simulink library "Renault_Library"  (Simulink 8.2)
settings: Solver=ode3  StartTime=0.0  StopTime=10.0  FixedStep=0.020  RelTol=1e-3
undefined symbols (not defined in the file or in its folder): ABC, AssystemProposal, b, chkval, CoeffA_C, CoeffB_C, CoeffMean_C, ConfTime_C, DiagDelay_C, DividePercent, FailureConfActive_C, FallingEdgeDuration_C, HighLevelThresholdVoltage_C, HighLevelVoltage_C, HighLevelVoltageTunable_C, InitValue_C, InputVoltRangeMax_C, InputVoltRangeMin_C, LowActiveContact_C, LowLevelThresholdVoltage_C, LowLevelVoltage_C, MaxOperatingVoltage_C, MinOperatingVoltage_C, mV2V_conversion, OUT1, OutOfRangeHigh_C, OutOfRangeLow_C, PinSupplySaturationMax, PinSupplySaturationMin, Res1Value_C, Res2Value_C, Res3Value_C, RisingEdgeDuration_C, RSA_CANSignalFailMonitoring, SampleTime_C, service14Table, service19Table, service22Table, service24Table, service28Table, service2ETable, service2FTable, service31Table, service87Table, SizeXStateDID2FTable, SizeXTable14, SizeXTable19, SizeXTable22, SizeXTable24, SizeXTable28, SizeXTable2E, SizeXTable2F, SizeXTable31, SizeXTable87, SizeYStateDID2FTable, SizeYTable14, SizeYTable19, SizeYTable22, SizeYTable24, SizeYTable28 ... (+22)
undefined symbols (not defined in the file): BatteryVoltage_ActivationThreshold_C, FrameStatus_AbsentValue_C, FrameStatus_ConfAbsentValue_C, MemThreshold_C, NAMEOFTHEMODEL_SAMPLE_TIME_CST, NbAbs1_C, NbConfAbs1_C, NbConfPres1_C, NbPres1_C, NOMDUSIGNAL_FaultConfTime_C, NOMDUSIGNAL_FaultRehabTime_C, NOMDUSIGNAL_switchvalue_C, NOMDUSIGNAL_unavailablevalue_C, PeriodFrame1_C, ResetTime_C, WakeUpTime_C
libraries: Renault_Library x112, simulink x75
(part 6 of 32)

== system Renault_Library/Services ECU/EEPROM ==
  note: EEPROM
  RSA_UpdateEEPROMData : Subsystem  Tag=[LIB]  mask(RSA_UpdateEEPROMData){}
      mask help:
      | Manage EEPROM data in model.
      |
      | Version : 01.00
      mask Help:
      | Manage EEPROM data in model.
      | 
      | Version : 01.00
      mask Display:
      | disp('EEPROM Management')
      | port_label('Input',1,'MODEL_EEPROM_DATA')
      | port_label('Input',2,'DATA_STORE_READ')
      | port_label('Output',1,'DATA_STORE_WRITE')

== system Renault_Library/Services ECU/HW Inputs ==
  note: DEFINED INPUTS
  note: FULLY TUNABLE INPUTS
  RSA_CHA8PRO : Subsystem  Tag=[LIB]  mask(RSA_CHA8PRO){HighLevelOutput_C=1, InitValue_C=0, SampleTime_C=1, Tstab_C=30}
      mask prompts: HighLevelOutput_C "State of output relay when high voltage (1 for active high, 0 for active low)"; InitValue_C "Initial Value of Output"; SampleTime_C "Ts: insert a sample time (ms)"; Tstab_C "Tstab : insert a stabilization waiting time (ms)"
      mask help:
      | CHA.8.PRO:
      | Input for active HIGH contact with medium current switch. Contact has a serial resistive protection.
      | Min Operating Voltage = 8 V
      | Max Operating Voltage = 16 V
      | High level input voltage >= 5.5V
      | Low level input voltage <= 2.5V
      | Unit of ports for voltage are in millivolt (mV)
      |
      | Version : 01.01
      mask init:
      | MinOperatingVoltage_C=8000;
      | MaxOperatingVoltage_C=16000; 
      | LowLevelThresholdVoltage_C=2500; 
      | HighLevelThresholdVoltage_C=5500; 
      | LowLevelOutput_C= not(HighLevelOutput_C);
      mask Help:
      | CHA.8.PRO:
      | Input for active HIGH contact with medium current switch. Contact has a serial resistive protection.
      | Min Operating Voltage = 8 V
      | Max Operating Voltage = 16 V
      | High level input voltage >= 5.5V
      | Low level input voltage <= 2.5V
      | Unit of ports for voltage are in millivolt (mV)
      | 
      | Version : 01.01
      mask Display: disp('CHA_8_PRO')
  RSA_CHB6 : Subsystem  Tag=[LIB]  mask(RSA_CHB6){HighLevelOutput_C=1, InitValue_C=0, SampleTime_C=1, Tstab_C=30}
      mask prompts: HighLevelOutput_C "State of output relay when high voltage (1 for active high, 0 for active low)"; InitValue_C "Initial value of Output"; SampleTime_C "Ts: insert a sample time (ms)"; Tstab_C "Tstab : insert a stabilization waiting time (ms)"
      mask help:
      | CHB.6:
      | Input for active HIGH contact shared between several consumers
      | Min Operating Voltage = 6 V
      | Max Operating Voltage = 16V
      | High level input voltage >= 5.5V
      | Low level input voltage <= 2.5V
      | Unit of ports for voltage are in millivolt (mV)
      |
      | Version : 01.01
      mask init:
      | MinOperatingVoltage_C=6000;%Min Operating Voltage. Range for which the considered input hardware stage should work correctly (mV)
      | MaxOperatingVoltage_C=16000; %Max Operating Voltage. Upper limit of range for which the considered input hardware stage should work correctly (mV)(normally 16V)
      | LowLevelThresholdVoltage_C=2500; %Low Level Threshold Voltage (mV)
      | HighLevelThresholdVoltage_C=5500; %High Level Threshold Voltage (mV)
      | 
      | LowLevelOutput_C= not(HighLevelOutput_C);
      | 
      | % InitValue_C=0; %Value of initial value when enabling
      mask Help:
      | CHB.6:
      | Input for active HIGH contact shared between several consumers
      | Min Operating Voltage = 6 V
      | Max Operating Voltage = 16V
      | High level input voltage >= 5.5V
      | Low level input voltage <= 2.5V
      | Unit of ports for voltage are in millivolt (mV)
      | 
      | Version : 01.01
      mask Display: disp('CHB_6')
  RSA_CHB6DAS : Subsystem  Tag=[LIB]  mask(RSA_CHB6DAS){HighLevelOutput_C=1, InitValue_C=0, SampleTime_C=1, Tstab_C=30}
      mask prompts: HighLevelOutput_C "State of output relay when high voltage (1 for active high, 0 for active low)"; InitValue_C "Initial value of Output"; SampleTime_C "Ts: insert a sample time (ms)"; Tstab_C "Tstab : insert a stabilization waiting time (ms)"
      mask help:
      | CHB.6.DAS:
      | Input for active HIGH contact with medium current switch. Signal shared between several consumers.
      | Min Operating Voltage = 6 V
      | Max Operating Voltage = 16V
      | High level input voltage >= 5.5V
      | Low level input voltage <= 2.5V
      | Unit of ports for voltage are in millivolt (mV)
      |
      | Version : 01.01
      mask init:
      | MinOperatingVoltage_C=6000;
      | MaxOperatingVoltage_C=16000; 
      | LowLevelThresholdVoltage_C=2500; 
      | HighLevelThresholdVoltage_C=5500; 
      | LowLevelOutput_C= not(HighLevelOutput_C);
      mask Help:
      | CHB.6.DAS:
      | Input for active HIGH contact with medium current switch. Signal shared between several consumers.
      | Min Operating Voltage = 6 V
      | Max Operating Voltage = 16V
      | High level input voltage >= 5.5V
      | Low level input voltage <= 2.5V
      | Unit of ports for voltage are in millivolt (mV)
      | 
      | Version : 01.01
      mask Display: disp('CHB_6_DAS')
  RSA_CHXX : Subsystem  Tag=[LIB]  mask(RSA_CHX.X){MinOperatingVoltage_C=0, MaxOperatingVoltage_C=0, LowLevelThresholdVoltage_C=0, HighLevelThresholdVoltage_C=0, HighLevelOutput_C=1, InitValue_C=0, SampleTime_C=1, Tstab_C=30}
      mask prompts: MinOperatingVoltage_C "Min Operating Voltage. Range for which the considered input hardware stage should work correctly (mV)"; MaxOperatingVoltage_C "Max Operating Voltage. Upper limit of range for which the considered input hardware stage should work correctly (mV)(normally 16V)"; LowLevelThresholdVoltage_C "Low Level Threshold Voltage (mV)"; HighLevelThresholdVoltage_C "High Level Threshold Voltage (mV)"; HighLevelOutput_C "State of output relay when high voltage (1 for active high, 0 for active low)"; InitValue_C "Initial value of Output"; SampleTime_C "Ts: insert a sample time (ms)"; Tstab_C "Tstab : insert a stabilization waiting time (ms)"
      mask help:
      | CHX.X:
      | Input for active HIGH contact
      | Unit of ports for voltage are in millivolt (mV)
      |
      | Version : 01.01
      mask init:
      | LowLevelOutput_C= not(HighLevelOutput_C);% InitValue_C=0; %Value of initial value when enabling
      mask Help:
      | CHX.X:
      | Input for active HIGH contact
      | Unit of ports for voltage are in millivolt (mV)
      | 
      | Version : 01.01
      mask Display: disp('CHX.X')
  RSA_CLA8LOCK : Subsystem  Tag=[LIB]  mask(RSA_CLA8LOCK){InitValue_C=0, Tstab_C=30, ConfTime_C=20, SampleTime_C=1, VonMax_C=4500, VonMin_C=500}
      mask prompts: InitValue_C "Initial Value of Output"; Tstab_C "Tstab : insert a stabilization waiting time (SoftwareFiltering) (ms)"; ConfTime_C "ConfTime : Confirmation Time in case of Failure (ms)"; SampleTime_C "SampleTime : SampleTime of block (ms)"; VonMax_C "Threshold (mV) above which a Resistive SC- failure is declared : Von_max"; VonMin_C "Threshold (mV) below which an SC- failure is declared :Von_min"
      mask help:
      | CLA.8.LOCK: Analog active low input with Interlock Switch.
      |
      | ECU input, active on low state. Link to one consumer.
      | Min Operating Voltage = 8 V
      | Max Operating Voltage = 16 V
      | Unit of ports for voltage are in millivolt (mV)
      |
      | Diagnosis:
      | Three failure states (SC+, SC-, OC), and two normal states (OKON and OKOFF) are possible.
      | The analogue thresholds to define these different states must take into account the battery voltage range.
      |
      |     State           SW Status               Diagnostic States
      |      4              V > Vonmax                  OC/SC+/OKOFF/Resistive SC-
      |      2          Vonmax > V > Vonmin             OKON
      |      1              V < Vonmin                  SC-
      |
      | Version : 01.01
      mask init:
      | MinOperatingVoltage_C=8000;
      | MaxOperatingVoltage_C=16000; 
      | State_InfVonMin_C=1;
      | State_Von_C=2;
      | State_SupVonMaxInfVoffmin_C=4;
      | State_Voff_C=4;
      | State_SupVoffMax_C=4;
      | VoffMax_C= 10000; %Masked parameter threshold (mV) above which an SC+ or OC failure is declared : Voff_max
      | VoffMin_C=5200; %Masked parameter threshold (mV) above which switch is OKOFF : Voff_min
      mask Help:
      | CLA.8.LOCK: Analog active low input with Interlock Switch.
      | 
      | ECU input, active on low state. Link to one consumer.
      | Min Operating Voltage = 8 V
      | Max Operating Voltage = 16 V
      | Unit of ports for voltage are in millivolt (mV)
      | 
      | Diagnosis:
      | Three failure states (SC+, SC-, OC), and two normal states (OKON and OKOFF) are possible.
      | The analogue thresholds to define these different states must take into account the battery voltage range.
      | 
      |     State           SW Status               Diagnostic States
      |      4              V > Vonmax                  OC/SC+/OKOFF/Resistive SC-
      |      2          Vonmax > V > Vonmin             OKON
      |      1              V < Vonmin                  SC-
      | 
      | Version : 01.01
      mask Display: disp('CLA_8_LOCK')
  RSA_CLA8MVAC : Subsystem  Tag=[LIB]  mask(RSA_CLA8MVAC){InitValue_C=0, Tstab_C=30, ConfTime_C=20, SampleTime_C=1, VoffMax_C=10000, VoffMin_C=5200, VonMax_C=1000}
      mask prompts: InitValue_C "Initial Value of Output"; Tstab_C "Tstab : insert a stabilization waiting time (SoftwareFiltering) (ms)"; ConfTime_C "ConfTime : Confirmation Time in case of Failure (ms)"; SampleTime_C "SampleTime : SampleTime of block (ms)"; VoffMax_C "Threshold (mV) above which an SC+ or OC failure is declared : Voff_max"; VoffMin_C "Threshold (mV) above which switch is OKOFF : Voff_min"; VonMax_C "Threshold (mV) above which a Resistive SC- failure is declared : Von_max"
      mask help:
      | CLA.8.MVAC: Analog input for active Low contact. Contact has a parallel resistor
      |
      | ECU input, active on low state.
      | Min Operating Voltage = 8 V
      | Max Operating Voltage = 16 V
      | Unit of ports for voltage are in millivolt (mV)
      |
      | Diagnosis:
      | Three failure states (SC+, SC-, OC), and two normal states (OKON and OKOFF) are possible.
      | The analogue thresholds to define these different states must take into account the battery voltage range.
      |
      |     State           SW Status               Diagnostic States
      |      5              V > Voffmax                 SC+ or OC
      |      4         Voffmax > V > Voffmin            OKOFF
      |      3         Voffmin > V > Vonmax             Resistive SC-
      |      2          Vonmax > V                      OKON or SC-
      |
      |
      | Version : 01.01
      mask init:
      | MinOperatingVoltage_C=8000;
      | MaxOperatingVoltage_C=16000; 
      | State_InfVonMin_C=2;
      | State_Von_C=2;
      | State_SupVonMaxInfVoffmin_C=3;
      | State_Voff_C=4;
      | State_SupVoffMax_C=5;
      | VonMin_C=0; %Masked parameter Threshold (mV) below which an SC- failure is declared :Von_min
      mask Help:
      | CLA.8.MVAC: Analog input for active Low contact. Contact has a parallel resistor
      | 
      | ECU input, active on low state.
      | Min Operating Voltage = 8 V
      | Max Operating Voltage = 16 V
      | Unit of ports for voltage are in millivolt (mV)
      | 
      | Diagnosis:
      | Three failure states (SC+, SC-, OC), and two normal states (OKON and OKOFF) are possible.
      | The analogue thresholds to define these different states must take into account the battery voltage range.
      | 
      |     State           SW Status               Diagnostic States
      |      5              V > Voffmax                 SC+ or OC
      |      4         Voffmax > V > Voffmin            OKOFF
      |      3         Voffmin > V > Vonmax             Resistive SC-
      |      2          Vonmax > V                      OKON or SC-
      | 
      | 
      | Version : 01.01
      mask Display: disp('CLA_8_MVAC')
  RSA_CLA8PRO : Subsystem  Tag=[LIB]  mask(RSA_CLA8PRO){HighLevelOutput_C=0, InitValue_C=0, SampleTime_C=1, Tstab_C=30}
      mask prompts: HighLevelOutput_C "State of output relay when high voltage (1 for active high, 0 for active low)"; InitValue_C "Initial value of Output"; SampleTime_C "Ts: insert a sample time (ms)"; Tstab_C "Tstab : insert a stabilization waiting time (ms)"
      mask help:
      | CLA.8.PRO:
      | Input for active LOW contact shared between several consumers. Contact has a serial resistive protection
      | Min Operating Voltage = 8 V
      | Max Operating Voltage = 16 V
      | High level input voltage >= 6V
      | Low level input voltage <= 4V
      | Unit of ports for voltage are in millivolt (mV)
      |
      | Version : 01.01
      mask init:
      | MinOperatingVoltage_C=8000;
      | MaxOperatingVoltage_C=16000;
      | LowLevelThresholdVoltage_C=4000; 
      | HighLevelThresholdVoltage_C=6000; 
      | LowLevelOutput_C= not(HighLevelOutput_C);
      mask Help:
      | CLA.8.PRO:
      | Input for active LOW contact shared between several consumers. Contact has a serial resistive protection
      | Min Operating Voltage = 8 V
      | Max Operating Voltage = 16 V
      | High level input voltage >= 6V
      | Low level input voltage <= 4V
      | Unit of ports for voltage are in millivolt (mV)
      | 
      | Version : 01.01
      mask Display: disp('CLA_8_PRO')
  RSA_CLA8SEAT : Subsystem  Tag=[LIB]  mask(RSA_CLA8SEAT){InitValue_C=0, Tstab_C=30, ConfTime_C=20, SampleTime_C=1, VoffMax_C=8000, VoffMin_C=4500, VonMax_C=3000, VonMin_C=500}
      mask prompts: InitValue_C "Initial Value of Output"; Tstab_C "Tstab : insert a stabilization waiting time (SoftwareFiltering) (ms)"; ConfTime_C "ConfTime : Confirmation Time in case of Failure (ms)"; SampleTime_C "SampleTime : SampleTime of block (ms)"; VoffMax_C "Threshold (mV) above which an SC+ or OC failure is declared : Voff_max"; VoffMin_C "Threshold (mV) above which switch is OKOFF : Voff_min"; VonMax_C "Threshold (mV) above which a Resistive SC- failure is declared : Von_max"; VonMin_C "Threshold (mV) below which an SC- failure is declared :Von_min"
      mask help:
      | CLA.8.SEAT: Analog active low input with seat sensor Switch.
      |
      | ECU input, active on low state. Link to one consumer.
      | Min Operating Voltage = 8 V
      | Max Operating Voltage = 16 V
      | Unit of ports for voltage are in millivolt (mV)
      |
      | Specific for SEAT
      |
      | Diagnosis:
      | Three failure states (SC+, SC-, OC), and two normal states (OKON and OKOFF) are possible.
      | The analogue thresholds to output these different states are tunable in diagnostic parameters tab.
      |
      |     State           SW Status               Diagnostic States
      |      5              V > Voffmax                 SC+ or OC
      |      4         Voffmax > V > Voffmin            OKOFF
      |      3         Voffmin > V > Vonmax             Resistive SC-
      |      2          Vonmax > V > Vonmin             OKON
      |      1              V < Vonmin                      SC-
      |
      | Version : 01.01
      mask init:
      | MinOperatingVoltage_C=8000;
      | MaxOperatingVoltage_C=16000;
      | State_InfVonMin_C=1;
      | State_Von_C=2;
      | State_SupVonMaxInfVoffmin_C=3;
      | State_Voff_C=4;
      | State_SupVoffMax_C=5;
      mask Help:
      | CLA.8.SEAT: Analog active low input with seat sensor Switch.
      | 
      | ECU input, active on low state. Link to one consumer.
      | Min Operating Voltage = 8 V
      | Max Operating Voltage = 16 V
      | Unit of ports for voltage are in millivolt (mV)
      | 
      | Specific for SEAT
      | 
      | Diagnosis:
      | Three failure states (SC+, SC-, OC), and two normal states (OKON and OKOFF) are possible.
      | The analogue thresholds to output these different states are tunable in diagnostic parameters tab.
      | 
      |     State           SW Status               Diagnostic States
      |      5              V > Voffmax                 SC+ or OC
      |      4         Voffmax > V > Voffmin            OKOFF
      |      3         Voffmin > V > Vonmax             Resistive SC-
      |      2          Vonmax > V > Vonmin             OKON
      |      1              V < Vonmin                      SC-
      | 
      | Version : 01.01
      mask Display: disp('CLA_8_SEAT')
  RSA_CLA8WAS : Subsystem  Tag=[LIB]  mask(RSA_CLA8WAS){HighLevelOutput_C=0, InitValue_C=0, SampleTime_C=1, Tstab_C=30}
      mask prompts: HighLevelOutput_C "High Level Output. State of output Relay"; InitValue_C "Initial Value of Output"; SampleTime_C "Ts: insert a sample time (ms)"; Tstab_C "Tstab : insert a stabilization waiting time (ms)"
      mask help:
      | CLA.8.WAS:
      | Input for active LOW input for Wiper auto stop switch. One single consumer
      | Min Operating Voltage = 8 V
      | Max Operating Voltage = 16 V
      | High level input voltage >= 7V
      | Low level input voltage <= 3V
      | Unit of ports for voltage are in millivolt (mV)
      |
      | Version : 01.01
      mask init:
      | MinOperatingVoltage_C=8000; 
      | MaxOperatingVoltage_C=16000; 
      | LowLevelThresholdVoltage_C=3000; 
      | HighLevelThresholdVoltage_C=7000; 
      | LowLevelOutput_C= not(HighLevelOutput_C);
      mask Help:
      | CLA.8.WAS:
      | Input for active LOW input for Wiper auto stop switch. One single consumer
      | Min Operating Voltage = 8 V
      | Max Operating Voltage = 16 V
      | High level input voltage >= 7V
      | Low level input voltage <= 3V
      | Unit of ports for voltage are in millivolt (mV)
      | 
      | Version : 01.01
      mask Display: disp('CLA_8_WAS')
  RSA_CLB6 : Subsystem  Tag=[LIB]  mask(RSA_CLB6){HighLevelOutput_C=0, InitValue_C=0, SampleTime_C=1, Tstab_C=30}
      mask prompts: HighLevelOutput_C "State of output relay when high voltage (1 for active high, 0 for active low)"; InitValue_C "Initial value of Output"; SampleTime_C "Ts: insert a sample time (ms)"; Tstab_C "Tstab : insert a stabilization waiting time (ms)"
      mask help:
      | CLB.6:
      | Input for active LOW contact shared between several consumers
      | Min Operating Voltage = 6 V
      | Max Operating Voltage = 16 V
      | High level input voltage >= 4.5V
      | Low level input voltage <= 2V
      | Unit of ports for voltage are in millivolt (mV)
      |
      | Version : 01.01
      mask init:
      | MinOperatingVoltage_C=6000;%Min Operating Voltage. Range for which the considered input hardware stage should work correctly (mV)
      | MaxOperatingVoltage_C=16000; %Max Operating Voltage. Upper limit of range for which the considered input hardware stage should work correctly (mV)(normally 16V)
      | 
      | LowLevelThresholdVoltage_C=2000; %Low Level Threshold Voltage (mV)
      | HighLevelThresholdVoltage_C=4500; %High Level Threshold Voltage (mV)
      | 
      | LowLevelOutput_C= not(HighLevelOutput_C);
      | 
      | % InitValue_C=0; %Value of initial value when enabling
      mask Help:
      | CLB.6:
      | Input for active LOW contact shared between several consumers
      | Min Operating Voltage = 6 V
      | Max Operating Voltage = 16 V
      | High level input voltage >= 4.5V
      | Low level input voltage <= 2V
      | Unit of ports for voltage are in millivolt (mV)
      | 
      | Version : 01.01
      mask Display: disp('CLB_6')
  RSA_CLB6DAS : Subsystem  Tag=[LIB]  mask(RSA_CLB6DAS){HighLevelOutput_C=0, InitValue_C=0, SampleTime_C=1, Tstab_C=30}
      mask prompts: HighLevelOutput_C "State of output relay when high voltage (1 for active high, 0 for active low)"; InitValue_C "Initial Value of Output"; SampleTime_C "Ts: insert a sample time (ms)"; Tstab_C "Tstab : insert a stabilization waiting time (ms)"
      mask help:
      | CLB.6.DAS:
      | Input for active LOW contact with medium current switch. Signal shared between several consumers
      | Min Operating Voltage = 6 V
      | Max Operating Voltage = 16 V
      | High level input voltage >= 4.5V
      | Low level input voltage <= 2.8V
      | Unit of ports for voltage are in millivolt (mV)
      |
      | Version : 01.01
      mask init:
      | MinOperatingVoltage_C=6000;
      | MaxOperatingVoltage_C=16000; 
      | LowLevelThresholdVoltage_C=2800; 
      | HighLevelThresholdVoltage_C=4500; 
      | LowLevelOutput_C= not(HighLevelOutput_C);
      mask Help:
      | CLB.6.DAS:
      | Input for active LOW contact with medium current switch. Signal shared between several consumers
      | Min Operating Voltage = 6 V
      | Max Operating Voltage = 16 V
      | High level input voltage >= 4.5V
      | Low level input voltage <= 2.8V
      | Unit of ports for voltage are in millivolt (mV)
      | 
      | Version : 01.01
      mask Display: disp('CLB_6_DAS')
  RSA_CLXX : Subsystem  Tag=[LIB]  mask(RSA_CLX.X){MinOperatingVoltage_C=0, MaxOperatingVoltage_C=0, HighLevelOutput_C=0, LowLevelThresholdVoltage_C=0, HighLevelThresholdVoltage_C=0, InitValue_C=0, SampleTime_C=1, Tstab_C=30}
      mask prompts: MinOperatingVoltage_C "Min Operating Voltage. Range for which the considered input hardware stage should work correctly (mV)"; MaxOperatingVoltage_C "Max Operating Voltage. Upper limit of range for which the considered input hardware stage should work correctly (mV)(normally 16V)"; HighLevelOutput_C "State of output relay when high voltage (1 for active high, 0 for active low)"; LowLevelThresholdVoltage_C "Low Level Threshold Voltage (mV)"; HighLevelThresholdVoltage_C "High Level Threshold Voltage (mV)"; InitValue_C "Initial value of Output"; SampleTime_C "Ts: insert a sample time (ms)"; Tstab_C "Tstab : insert a stabilization waiting time (ms)"
      mask help:
      | CLX.X:
      | Input for active LOW contact
      | Unit of ports for voltage are in millivolt (mV)
      |
      | Version : 01.01
      mask init:
      | LowLevelOutput_C= not(HighLevelOutput_C);
      mask Help:
      | CLX.X:
      | Input for active LOW contact
      | Unit of ports for voltage are in millivolt (mV)
      | 
      | Version : 01.01
      mask Display: disp('CLX.X')
  RSA_IHB6 : Subsystem  Tag=[LIB]  mask(RSA_IHB6){HighLevelOutput_C=1, InitValue_C=0, SampleTime_C=1, Tstab_C=30}
      mask prompts: HighLevelOutput_C "State of output relay when high voltage (1 for active high, 0 for active low)"; InitValue_C "Initial value of Output"; SampleTime_C "Ts: insert a sample time (ms)"; Tstab_C "Tstab : insert a stabilization waiting time (ms)"
      mask help:
      | IHB.6:
      | Active HIGH input. Several consumers
      | Min Operating Voltage = 6 V
      | Max Operating Voltage = 16V
      | High level input voltage >= 3.5V
      | Low level input voltage <= 1.5V
      | Unit of ports for voltage are in millivolt (mV)
      |
      | Version : 01.01
      mask init:
      | MinOperatingVoltage_C=6000;
      | MaxOperatingVoltage_C=16000; 
      | LowLevelThresholdVoltage_C=1500; 
      | HighLevelThresholdVoltage_C=3500; 
      | LowLevelOutput_C= not(HighLevelOutput_C);
      mask Help:
      | IHB.6:
      | Active HIGH input. Several consumers
      | Min Operating Voltage = 6 V
      | Max Operating Voltage = 16V
      | High level input voltage >= 3.5V
      | Low level input voltage <= 1.5V
      | Unit of ports for voltage are in millivolt (mV)
      | 
      | Version : 01.01
      mask Display: disp('IHB_6')
  RSA_IHB6LED : Subsystem  Tag=[LIB]  mask(RSA_IHB6LED){HighLevelOutput_C=1, InitValue_C=0, SampleTime_C=1, Tstab_C=30}
      mask prompts: HighLevelOutput_C "State of output relay when high voltage (1 for active high, 0 for active low)"; InitValue_C "Initial value of Output"; SampleTime_C "Ts: insert a sample time (ms)"; Tstab_C "Tstab : insert a stabilization waiting time (ms)"
      mask help:
      | IHB.6.LED:
      | Active HIGH LED input. Several consumers
      | Min Operating Voltage = 6 V
      | Max Operating Voltage = 16V
      | High level input voltage >= 3.5V
      | Low level input voltage <= 1.5V
      | Unit of ports for voltage are in millivolt (mV)
      |
      | Version : 01.01
      mask init:
      | MinOperatingVoltage_C=6000;
      | MaxOperatingVoltage_C=16000; 
      | LowLevelThresholdVoltage_C=1500; 
      | HighLevelThresholdVoltage_C=3500; 
      | LowLevelOutput_C= not(HighLevelOutput_C);
      mask Help:
      | IHB.6.LED:
      | Active HIGH LED input. Several consumers
      | Min Operating Voltage = 6 V
      | Max Operating Voltage = 16V
      | High level input voltage >= 3.5V
      | Low level input voltage <= 1.5V
      | Unit of ports for voltage are in millivolt (mV)
      | 
      | Version : 01.01
      mask Display: disp('IHB_6_LED')
  RSA_IHXX : Subsystem  Tag=[LIB]  mask(RSA_IHX.X){MinOperatingVoltage_C=0, MaxOperatingVoltage_C=0, LowLevelThresholdVoltage_C=0, HighLevelThresholdVoltage_C=0, HighLevelOutput_C=1, InitValue_C=0, SampleTime_C=1, Tstab_C=30}
      mask prompts: MinOperatingVoltage_C "Min Operating Voltage. Range for which the considered input hardware stage should work correctly (mV)"; MaxOperatingVoltage_C "Max Operating Voltage. Upper limit of range for which the considered input hardware stage should work correctly (mV)(normally 16V)"; LowLevelThresholdVoltage_C "Low Level Threshold Voltage (mV)"; HighLevelThresholdVoltage_C "High Level Threshold Voltage (mV)"; HighLevelOutput_C "State of output relay when high voltage (1 for active high, 0 for active low)"; InitValue_C "Initial value of Output"; SampleTime_C "Ts: insert a sample time (ms)"; Tstab_C "Tstab : insert a stabilization waiting time (ms)"
      mask help:
      | IHX.X:
      | Input for active HIGH contact
      | Unit of ports for voltage are in millivolt (mV)
      |
      | Version : 01.01
      mask init:
      | LowLevelOutput_C= not(HighLevelOutput_C);
      mask Help:
      | IHX.X:
      | Input for active HIGH contact
      | Unit of ports for voltage are in millivolt (mV)
      | 
      | Version : 01.01
      mask Display: disp('IHX.X')
  RSA_ILB6 : Subsystem  Tag=[LIB]  mask(RSA_ILB6){HighLevelOutput_C=0, InitValue_C=0, SampleTime_C=1, Tstab_C=30}
      mask prompts: HighLevelOutput_C "State of output relay when high voltage (1 for active high, 0 for active low)"; InitValue_C "Initial Value of Output"; SampleTime_C "Ts: insert a sample time (ms)"; Tstab_C "Tstab : insert a stabilization waiting time (ms)"
      mask help:
      | ILB.6:
      | Active LOW input. Several consumers
      | Min Operating Voltage = 6 V
      | Max Operating Voltage = 16 V
      | High level input voltage >= 4.5V
      | Low level input voltage <= 2V
      | Unit of ports for voltage are in millivolt (mV)
      |
      | Version : 01.01
      mask init:
      | MinOperatingVoltage_C=6000;
      | MaxOperatingVoltage_C=16000; 
      | LowLevelThresholdVoltage_C=2000; 
      | HighLevelThresholdVoltage_C=4500; 
      | LowLevelOutput_C= not(HighLevelOutput_C);
      mask Help:
      | ILB.6:
      | Active LOW input. Several consumers
      | Min Operating Voltage = 6 V
      | Max Operating Voltage = 16 V
      | High level input voltage >= 4.5V
      | Low level input voltage <= 2V
      | Unit of ports for voltage are in millivolt (mV)
      | 
      | Version : 01.01
      mask Display: disp('ILB_6')
  RSA_ILB6LED : Subsystem  Tag=[LIB]  mask(RSA_ILB6LED){HighLevelOutput_C=0, InitValue_C=0, SampleTime_C=1, Tstab_C=30}
      mask prompts: HighLevelOutput_C "State of output relay when high voltage (1 for active high, 0 for active low)"; InitValue_C "Initial value of Output"; SampleTime_C "Ts: insert a sample time (ms)"; Tstab_C "Tstab : insert a stabilization waiting time (ms)"
      mask help:
      | ILB.6.LED:
      | Active LOW LED input. Several consumers
      | Min Operating Voltage = 6 V
      | Max Operating Voltage = 16 V
      | High level input voltage >= 4.5V
      | Low level input voltage <= 2V
      | Unit of ports for voltage are in millivolt (mV)
      |
      | Version : 01.01
      mask init:
      | MinOperatingVoltage_C=6000;
      | MaxOperatingVoltage_C=16000; 
      | LowLevelThresholdVoltage_C=2000; 
      | HighLevelThresholdVoltage_C=4500; 
      | LowLevelOutput_C= not(HighLevelOutput_C);
      mask Help:
      | ILB.6.LED:
      | Active LOW LED input. Several consumers
      | Min Operating Voltage = 6 V
      | Max Operating Voltage = 16 V
      | High level input voltage >= 4.5V
      | Low level input voltage <= 2V
      | Unit of ports for voltage are in millivolt (mV)
      | 
      | Version : 01.01
      mask Display: disp('ILB_6_LED')
  RSA_ILXX : Subsystem  Tag=[LIB]  mask(RSA_ILX.X){MinOperatingVoltage_C=0, MaxOperatingVoltage_C=0, LowLevelThresholdVoltage_C=0, HighLevelThresholdVoltage_C=0, HighLevelOutput_C=0, InitValue_C=0, SampleTime_C=1, Tstab_C=30}
      mask prompts: MinOperatingVoltage_C "Min Operating Voltage. Range for which the considered input hardware stage should work correctly (mV)"; MaxOperatingVoltage_C "Max Operating Voltage. Upper limit of range for which the considered input hardware stage should work correctly (mV)(normally 16V)"; LowLevelThresholdVoltage_C "Low Level Threshold Voltage (mV)"; HighLevelThresholdVoltage_C "High Level Threshold Voltage (mV)"; HighLevelOutput_C "State of output relay when high voltage (1 for active high, 0 for active low)"; InitValue_C "Initial Value of Output"; SampleTime_C "Ts: insert a sample time (ms)"; Tstab_C "Tstab : insert a stabilization waiting time (ms)"
      mask help:
      | ILX.X:
      | Input for active LOW contact
      | Unit of ports for voltage are in millivolt (mV)
      |
      | Version : 01.01
      mask init:
      | LowLevelOutput_C= not(HighLevelOutput_C);
      mask Help:
      | ILX.X:
      | Input for active LOW contact
      | Unit of ports for voltage are in millivolt (mV)
      | 
      | Version : 01.01
      mask Display: disp('ILX.X')
  RSA_IZB5TTL : Subsystem  Tag=[LIB]  mask(RSA_IZB5TTL){HighLevelOutput_C=1, InitValue_C=0, SampleTime_C=1, Tstab_C=30}
      mask prompts: HighLevelOutput_C "State of output relay when high voltage (1 for active high, 0 for active low)"; InitValue_C "Initial Value of Output"; SampleTime_C "Ts: insert a sample time (ms)"; Tstab_C "Tstab : insert a stabilization waiting time (ms)"
      mask help:
      | IZB.5.TTL:
      | Input stage for Push-Pull Output
      | Min Operating Voltage = 4.5 V
      | Max Operating Voltage = 5.5 V
      | High level input voltage >= 2V
      | Low level input voltage <= 0.8V
      | Unit of ports for voltage are in millivolt (mV)
      |
      | Version : 01.01
      mask init:
      | MinOperatingVoltage_C=4500;
      | MaxOperatingVoltage_C=5500; 
      | LowLevelThresholdVoltage_C=800; 
      | HighLevelThresholdVoltage_C=2000; 
      | LowLevelOutput_C= not(HighLevelOutput_C);
      mask Help:
      | IZB.5.TTL:
      | Input stage for Push-Pull Output
      | Min Operating Voltage = 4.5 V
      | Max Operating Voltage = 5.5 V
      | High level input voltage >= 2V
      | Low level input voltage <= 0.8V
      | Unit of ports for voltage are in millivolt (mV)
      | 
      | Version : 01.01
      mask Display: disp('IZB_5_TTL')
  RSA_RHEO9 : Subsystem  Tag=[LIB]  mask(RSA_RHEO9){InitValue_C=0, OutOfRangeLow_C=0, OutOfRangeHigh_C=0, SampleTime_C=1, Tstab_C=30, CoeffA_C=1, CoeffB_C=0, CoeffMean_C=1}
      mask prompts: InitValue_C "Initial Value of Output"; OutOfRangeLow_C "Value of output when input is below the range"; OutOfRangeHigh_C "Value of output when input is above the range"; SampleTime_C "SampleTime: insert a sample time (ms)"; Tstab_C "Tstab : insert a stabilization waiting time (ms)"; CoeffA_C "Slope: A Coefficient"; CoeffB_C "Offset: B Coefficient"; CoeffMean_C "Mean : M Coefficient"
      mask help:
      | RHEOSTAT.9:
      | Analogue input for shared resistive sensor.
      | Min Operating Voltage = 9 V
      | Max Operating Voltage = 16V
      |
      | Input Voltage Range: 0 <= In <= 9.5V
      | OutVoltage= InputVoltage x A + B
      | MeanOutVoltage = OutVoltage / M
      |
      | Version : 01.01
      mask init:
      | MinOperatingVoltage_C=9000;
      | MaxOperatingVoltage_C=16000;
      | InputVoltRangeMin_C=0; 
      | InputVoltRangeMax_C=9500;
      | 
      | testMean = CoeffMean_C;
      | if testMean < 1
      |     CoeffMean_C = 1;
      | elseif testMean > 10
      |     CoeffMean_C = 10;
      | elseif ~isinteger(testMean)
      |     CoeffMean_C = floor(testMean);
      | end
      | assignin('base','CoeffMean_C',CoeffMean_C);
      mask Help:
      | RHEOSTAT.9:
      | Analogue input for shared resistive sensor.
      | Min Operating Voltage = 9 V
      | Max Operating Voltage = 16V
      | 
      | Input Voltage Range: 0 <= In <= 9.5V
      | OutVoltage= InputVoltage x A + B
      | MeanOutVoltage = OutVoltage / M
      | 
      | Version : 01.01
      mask Display: disp('RHEOSTAT_9')
  RSA_VMEAS6 : Subsystem  Tag=[LIB]  mask(RSA_VMEAS6){InitValue_C=0, OutOfRangeLow_C=0, OutOfRangeHigh_C=0, SampleTime_C=1, Tstab_C=30, CoeffA_C=1, CoeffB_C=0, CoeffMean_C=1}
      mask prompts: InitValue_C "Initial Value of Output"; OutOfRangeLow_C "Value of output when input is below the range"; OutOfRangeHigh_C "Value of output when input is above the range"; SampleTime_C "SampleTime: insert a sample time (ms)"; Tstab_C "Tstab : insert a stabilization waiting time (ms)"; CoeffA_C "Slope: A Coefficient"; CoeffB_C "Offset: B Coeffcient"; CoeffMean_C "Mean : M Coefficient"
      mask help:
      | VOLTMEAS.6:
      | Analogue input for battery voltage measurement.
      | Min Operating Voltage = 6 V
      | Max Operating Voltage = 16 V
      |
      | Input Voltage Range: 0 <= In <= 24V
      | OutVoltage= InputVoltage x A + B
      | MeanOutVoltage = OutVoltage / M
      |
      | Version : 01.01
      mask init:
      | MinOperatingVoltage_C=6000;
      | MaxOperatingVoltage_C=16000; 
      | InputVoltRangeMin_C=0; 
      | InputVoltRangeMax_C=24000;
      | 
      | testMean = CoeffMean_C;
      | if testMean < 1
      |     CoeffMean_C = 1;
      | elseif testMean > 10
      |     CoeffMean_C = 10;
      | elseif ~isinteger(testMean)
      |     CoeffMean_C = floor(testMean);
      | end
      | assignin('base','CoeffMean_C',CoeffMean_C);
      mask Help:
      | VOLTMEAS.6:
      | Analogue input for battery voltage measurement.
      | Min Operating Voltage = 6 V
      | Max Operating Voltage = 16 V
      | 
      | Input Voltage Range: 0 <= In <= 24V
      | OutVoltage= InputVoltage x A + B
      | MeanOutVoltage = OutVoltage / M
      | 
      | Version : 01.01
      mask Display: disp('VOLTMEAS_6')
